simulink model "TosAeb"  (R2021a)
settings: Solver=FixedStepDiscrete  StartTime=0.0  StopTime=inf  FixedStep=0.01  RelTol=1e-3
undefined symbols (not defined in the file or in its folder): ACCModSts, AccSts1, AEB_ObjectMovingOrientation, AEB_ObjectMovingState, CN_BTCActive, CN_CurrentGearR, CN_CurrentGearR_DHT, CN_PTCActive, CN_VDCActive, CN_VSC_EspOff, CN_VSC_FCW_AEB_Error, CN_VSC_FCW_AEB_NoDispay, CN_VSC_FCW_AEB_PerfmDgdtn, CN_VSC_FCW_AEB_TempUnAvlbl, CN_VSC_VehStandstill, CNaccelAvoidMax, CNaccelAvoidMin, CNbrakePedalPress, CNcomfortStrLeftAccelMax, CNcomfortStrLeftAccelMin, CNcomfortStrRightAccelMax, CNcomfortStrRightAccelMin, CNDrvDoorOpen, CNDrvModeEco, CNDrvModeNorm, CNDrvModeOther, CNDrvModeSport, CNDrvSBRreminder, CNNull, CNobjInvalidID, CNPassDoorOpen, CNPassSBRreminder, CNPEBSAccelNegInf, CNPEBSAccelNull, CNPEBSTimeInf, CNPEBSTimeNull, CNSTOPMAX, CNSTOPNULL, CNsubjWidth, CNSwtOFF, CNSwtON, CNTTCMAX, CNTTCNULL, CNVehConfigAEBCycNone, CNVehConfigAEBNone, CNVehConfigAEBPedNone, CNVehConfigFCWNone, CNVehConfigJANone, CollisionSeverity, FALSE, FCWSnvty, JACycObjCalcInfoDefaultParams, JAPedObjCalcInfoDefaultParams, JAVehObjCalcInfoDefaultParams, latCycObjCalcInfoDefaultParams, latPedObjCalcInfoDefaultParams, longObjCalcInfoDefaultParams, ModSts, movingStatus, ObjectType ... (+1112)
libraries: simulink x3031, AEBSFunctionLib x50, Ta_Library x33, AEBSStateMachineLib x23, Mylibrary x12, simulink_extras x7, ObjSelect_lib x3, dspstat3 x2
(part 34 of 102)

== system TosAeb/FcwAebJa_Step_sys/ALG_AEBSystem/ALG_AEBController/AEB_VehicleStateCalcApp/JaSuppAborCondtionCalc/JAVehPrefillSuppAborConditonCalc/VehStateCalcJAPrefillAborCalc ==
  AEB_JAResp : Inport
  FCW_AEB_JAFctSts : Inport  Port=2
  vehAssociatedSysStateSuppCond : Inport  Port=3
  vehUnstableStateFlg : Inport  Port=4
  Compare To Constant : simulink/Logic and Bit Operations/Compare To Constant  relop===  const=CNSwtOFF  OutDataTypeStr=boolean
  Compare To Constant2 : simulink/Logic and Bit Operations/Compare To Constant  relop===  const=TRUE  OutDataTypeStr=boolean
  Compare To Constant3 : simulink/Logic and Bit Operations/Compare To Constant  relop===  const=TRUE  OutDataTypeStr=boolean
  Compare To Constant4 : simulink/Logic and Bit Operations/Compare To Constant  relop===  const=CN_VSC_FCW_AEB_Error  OutDataTypeStr=boolean
  Compare To Constant8 : simulink/Logic and Bit Operations/Compare To Constant  relop===  const=CN_VSC_FCW_AEB_PerfmDgdtn  OutDataTypeStr=boolean
  Compare To Constant9 : simulink/Logic and Bit Operations/Compare To Constant  relop===  const=CN_VSC_FCW_AEB_TempUnAvlbl  OutDataTypeStr=boolean
  Logical Operator : Logic  Operator=OR  Inputs=3  AllPortsSameDT=off  OutDataTypeStr=boolean
  Logical Operator1 : Logic  Operator=OR  Inputs=4  AllPortsSameDT=off  OutDataTypeStr=boolean
  VSCJAVehPrefillAborCond : Outport
  wires:
  Compare To Constant3/1 -> Logical Operator1/4
  Compare To Constant2/1 -> Logical Operator1/3
  AEB_JAResp/1 -> Compare To Constant/1
  Compare To Constant/1 -> Logical Operator1/1
  vehAssociatedSysStateSuppCond/1 -> Compare To Constant2/1
  Logical Operator1/1 -> VSCJAVehPrefillAborCond/1
  vehUnstableStateFlg/1 -> Compare To Constant3/1
  Compare To Constant4/1 -> Logical Operator/1
  Compare To Constant8/1 -> Logical Operator/2
  Compare To Constant9/1 -> Logical Operator/3
  Logical Operator/1 -> Logical Operator1/2
  FCW_AEB_JAFctSts/1 -> Compare To Constant9/1, Compare To Constant8/1, Compare To Constant4/1

== system TosAeb/FcwAebJa_Step_sys/ALG_AEBSystem/ALG_AEBController/AEB_VehicleStateCalcApp/JaSuppAborCondtionCalc/JAVehPrefillSuppAborConditonCalc/VehStateCalcJAPrefillSuppCalc ==
  AEB_JAResp : Inport
  FCW_AEB_JAFctSts : Inport  Port=2
  vehUnstableStateFlg : Inport  Port=3
  vehAssociatedSysStateSuppCond : Inport  Port=4
  "VehConfigAEBJA " : Inport  Port=5
  blockTimeBrakeReady : Inport  Port=6
  Compare To Constant : simulink/Logic and Bit Operations/Compare To Constant  relop===  const=CNSwtOFF  OutDataTypeStr=boolean
  Compare To Constant2 : simulink/Logic and Bit Operations/Compare To Constant  relop===  const=CN_VSC_FCW_AEB_Error  OutDataTypeStr=boolean
  Compare To Constant3 : simulink/Logic and Bit Operations/Compare To Constant  relop===  const=CNVehConfigJANone  OutDataTypeStr=boolean
  Compare To Constant4 : simulink/Logic and Bit Operations/Compare To Constant  relop=>  const=CNNull  OutDataTypeStr=boolean
  Compare To Constant5 : simulink/Logic and Bit Operations/Compare To Constant  relop===  const=TRUE  OutDataTypeStr=boolean
  Compare To Constant6 : simulink/Logic and Bit Operations/Compare To Constant  relop===  const=TRUE  OutDataTypeStr=boolean
  Compare To Constant7 : simulink/Logic and Bit Operations/Compare To Constant  relop===  const=CNSwtOFF  OutDataTypeStr=boolean
  Compare To Constant8 : simulink/Logic and Bit Operations/Compare To Constant  relop===  const=CN_VSC_FCW_AEB_PerfmDgdtn  OutDataTypeStr=boolean
  Compare To Constant9 : simulink/Logic and Bit Operations/Compare To Constant  relop===  const=CN_VSC_FCW_AEB_TempUnAvlbl  OutDataTypeStr=boolean
  Constant : Constant  Value=P_AEB_InterSwtSts4JAVehPrefill
  Logical Operator : Logic  Operator=OR  Inputs=3  AllPortsSameDT=off  OutDataTypeStr=boolean
  Logical Operator1 : Logic  Operator=OR  Inputs=6  AllPortsSameDT=off  OutDataTypeStr=boolean
  Terminator : Terminator
  VSCJAVehPrefillSuppCond : Outport
  wires:
  Compare To Constant7/1 -> Logical Operator1/6
  Constant/1 -> Compare To Constant7/1
  Compare To Constant6/1 -> Logical Operator1/3
  Compare To Constant5/1 -> Logical Operator1/4
  Compare To Constant4/1 -> Terminator/1
  "VehConfigAEBJA "/1 -> Compare To Constant3/1
  Logical Operator1/1 -> VSCJAVehPrefillSuppCond/1
  vehUnstableStateFlg/1 -> Compare To Constant6/1
  blockTimeBrakeReady/1 -> Compare To Constant4/1
  vehAssociatedSysStateSuppCond/1 -> Compare To Constant5/1
  Compare To Constant3/1 -> Logical Operator1/5
  Compare To Constant/1 -> Logical Operator1/1
  AEB_JAResp/1 -> Compare To Constant/1
  Compare To Constant2/1 -> Logical Operator/1
  Compare To Constant8/1 -> Logical Operator/2
  Compare To Constant9/1 -> Logical Operator/3
  Logical Operator/1 -> Logical Operator1/2
  FCW_AEB_JAFctSts/1 -> Compare To Constant9/1, Compare To Constant8/1, Compare To Constant2/1

== system TosAeb/FcwAebJa_Step_sys/ALG_AEBSystem/ALG_AEBController/AEB_VehicleStateCalcApp/PedSuppAborCondtionCalc/PedABASuppAborConditonCalc/VehStateCalcPedABAAborCalc ==
  AEB_PedResp : Inport
  FCW_AEB_PedFctSts : Inport  Port=2
  Compare To Constant : simulink/Logic and Bit Operations/Compare To Constant  relop===  const=CNSwtOFF  OutDataTypeStr=boolean
  Compare To Constant1 : simulink/Logic and Bit Operations/Compare To Constant  relop===  const=CN_VSC_FCW_AEB_Error  OutDataTypeStr=boolean
  Compare To Constant2 : simulink/Logic and Bit Operations/Compare To Constant  relop===  const=CN_VSC_FCW_AEB_PerfmDgdtn  OutDataTypeStr=boolean
  Compare To Constant8 : simulink/Logic and Bit Operations/Compare To Constant  relop===  const=CN_VSC_FCW_AEB_TempUnAvlbl  OutDataTypeStr=boolean
  Logical Operator : Logic  Operator=OR  Inputs=3  AllPortsSameDT=off  OutDataTypeStr=boolean
  Logical Operator1 : Logic  Operator=OR  AllPortsSameDT=off  OutDataTypeStr=boolean
  VSCPedABAAborCond : Outport
  wires:
  FCW_AEB_PedFctSts/1 -> Compare To Constant1/1, Compare To Constant2/1, Compare To Constant8/1
  Compare To Constant8/1 -> Logical Operator/3
  Compare To Constant1/1 -> Logical Operator/1
  Compare To Constant2/1 -> Logical Operator/2
  AEB_PedResp/1 -> Compare To Constant/1
  Compare To Constant/1 -> Logical Operator1/1
  Logical Operator/1 -> Logical Operator1/2
  Logical Operator1/1 -> VSCPedABAAborCond/1

== system TosAeb/FcwAebJa_Step_sys/ALG_AEBSystem/ALG_AEBController/AEB_VehicleStateCalcApp/PedSuppAborCondtionCalc/PedABASuppAborConditonCalc/VehStateCalcPedABASuppCalc ==
  VSCAEB_PedResp : Inport
  FCW_AEB_PedFctSts : Inport  Port=2
  vehUnstableStateFlg : Inport  Port=3
  vehAssociatedSysStateSuppCond : Inport  Port=4
  "vehConfigAEBPed " : Inport  Port=5
  blockTimeBrakeReady : Inport  Port=6
  Compare To Constant : simulink/Logic and Bit Operations/Compare To Constant  relop===  const=CNSwtOFF  OutDataTypeStr=boolean
  Compare To Constant1 : simulink/Logic and Bit Operations/Compare To Constant  relop===  const=CN_VSC_FCW_AEB_Error  OutDataTypeStr=boolean
  Compare To Constant2 : simulink/Logic and Bit Operations/Compare To Constant  relop===  const=TRUE  OutDataTypeStr=boolean
  Compare To Constant3 : simulink/Logic and Bit Operations/Compare To Constant  relop===  const=CNVehConfigAEBPedNone  OutDataTypeStr=boolean
  Compare To Constant4 : simulink/Logic and Bit Operations/Compare To Constant  relop=>  const=CNNull  OutDataTypeStr=boolean
  Compare To Constant5 : simulink/Logic and Bit Operations/Compare To Constant  relop===  const=TRUE  OutDataTypeStr=boolean
  Compare To Constant6 : simulink/Logic and Bit Operations/Compare To Constant  relop===  const=CN_VSC_FCW_AEB_PerfmDgdtn  OutDataTypeStr=boolean
  Compare To Constant7 : simulink/Logic and Bit Operations/Compare To Constant  relop===  const=CNSwtOFF  OutDataTypeStr=boolean
  Compare To Constant8 : simulink/Logic and Bit Operations/Compare To Constant  relop===  const=CN_VSC_FCW_AEB_TempUnAvlbl  OutDataTypeStr=boolean
  Constant : Constant  Value=P_AEB_InterSwtSts4PedABA
  Logical Operator : Logic  Operator=OR  Inputs=3  AllPortsSameDT=off  OutDataTypeStr=boolean
  Logical Operator1 : Logic  Operator=OR  Inputs=6  AllPortsSameDT=off  OutDataTypeStr=boolean
  Terminator : Terminator
  VSCPedABASuppCond : Outport
  wires:
  FCW_AEB_PedFctSts/1 -> Compare To Constant1/1, Compare To Constant6/1, Compare To Constant8/1
  Logical Operator/1 -> Logical Operator1/2
  Compare To Constant8/1 -> Logical Operator/3
  Compare To Constant1/1 -> Logical Operator/1
  Compare To Constant6/1 -> Logical Operator/2
  Compare To Constant7/1 -> Logical Operator1/6
  Constant/1 -> Compare To Constant7/1
  Compare To Constant5/1 -> Logical Operator1/4
  Compare To Constant2/1 -> Logical Operator1/3
  Compare To Constant4/1 -> Terminator/1
  "vehConfigAEBPed "/1 -> Compare To Constant3/1
  Logical Operator1/1 -> VSCPedABASuppCond/1
  vehUnstableStateFlg/1 -> Compare To Constant2/1
  blockTimeBrakeReady/1 -> Compare To Constant4/1
  vehAssociatedSysStateSuppCond/1 -> Compare To Constant5/1
  Compare To Constant3/1 -> Logical Operator1/5
  Compare To Constant/1 -> Logical Operator1/1
  VSCAEB_PedResp/1 -> Compare To Constant/1

== system TosAeb/FcwAebJa_Step_sys/ALG_AEBSystem/ALG_AEBController/AEB_VehicleStateCalcApp/PedSuppAborCondtionCalc/PedAEBfullSuppAborConditonCalc/VehStateCalcPedAEBfullAborCalc ==
  AEB_PedResp : Inport
  FCW_AEB_PedFctSts : Inport  Port=2
  vehAssociatedSysStateSuppCond : Inport  Port=3
  vehUnstableStateFlg : Inport  Port=4
  Compare To Constant : simulink/Logic and Bit Operations/Compare To Constant  relop===  const=CNSwtOFF  OutDataTypeStr=boolean
  Compare To Constant2 : simulink/Logic and Bit Operations/Compare To Constant  relop===  const=TRUE  OutDataTypeStr=boolean
  Compare To Constant3 : simulink/Logic and Bit Operations/Compare To Constant  relop===  const=TRUE  OutDataTypeStr=boolean
  Compare To Constant4 : simulink/Logic and Bit Operations/Compare To Constant  relop===  const=CN_VSC_FCW_AEB_Error  OutDataTypeStr=boolean
  Compare To Constant5 : simulink/Logic and Bit Operations/Compare To Constant  relop===  const=CN_VSC_FCW_AEB_PerfmDgdtn  OutDataTypeStr=boolean
  Compare To Constant8 : simulink/Logic and Bit Operations/Compare To Constant  relop===  const=CN_VSC_FCW_AEB_TempUnAvlbl  OutDataTypeStr=boolean
  Logical Operator : Logic  Operator=OR  Inputs=3  AllPortsSameDT=off  OutDataTypeStr=boolean
  Logical Operator1 : Logic  Operator=OR  Inputs=4  AllPortsSameDT=off  OutDataTypeStr=boolean
  VSCPedAEBfullAborCond : Outport
  wires:
  FCW_AEB_PedFctSts/1 -> Compare To Constant4/1, Compare To Constant5/1, Compare To Constant8/1
  Compare To Constant4/1 -> Logical Operator/1
  Compare To Constant5/1 -> Logical Operator/2
  Compare To Constant8/1 -> Logical Operator/3
  Compare To Constant3/1 -> Logical Operator1/4
  Compare To Constant2/1 -> Logical Operator1/3
  vehUnstableStateFlg/1 -> Compare To Constant3/1
  Logical Operator1/1 -> VSCPedAEBfullAborCond/1
  Logical Operator/1 -> Logical Operator1/2
  vehAssociatedSysStateSuppCond/1 -> Compare To Constant2/1
  Compare To Constant/1 -> Logical Operator1/1
  AEB_PedResp/1 -> Compare To Constant/1

== system TosAeb/FcwAebJa_Step_sys/ALG_AEBSystem/ALG_AEBController/AEB_VehicleStateCalcApp/PedSuppAborCondtionCalc/PedAEBfullSuppAborConditonCalc/VehStateCalcPedAEBfullSuppCalc ==
  AEB_PedResp : Inport
  FCW_AEB_PedFctSts : Inport  Port=2
  vehUnstableStateFlg : Inport  Port=3
  vehAssociatedSysStateSuppCond : Inport  Port=4
  "vehConfigAEBPed " : Inport  Port=5
  blockTimeBrake : Inport  Port=6
  Compare To Constant : simulink/Logic and Bit Operations/Compare To Constant  relop===  const=CNSwtOFF  OutDataTypeStr=boolean
  Compare To Constant1 : simulink/Logic and Bit Operations/Compare To Constant  relop===  const=CN_VSC_FCW_AEB_Error  OutDataTypeStr=boolean
  Compare To Constant2 : simulink/Logic and Bit Operations/Compare To Constant  relop===  const=TRUE  OutDataTypeStr=boolean
  Compare To Constant3 : simulink/Logic and Bit Operations/Compare To Constant  relop===  const=CNVehConfigAEBPedNone  OutDataTypeStr=boolean
  Compare To Constant4 : simulink/Logic and Bit Operations/Compare To Constant  relop=>  const=CNNull  OutDataTypeStr=boolean
  Compare To Constant5 : simulink/Logic and Bit Operations/Compare To Constant  relop===  const=TRUE  OutDataTypeStr=boolean
  Compare To Constant6 : simulink/Logic and Bit Operations/Compare To Constant  relop===  const=CN_VSC_FCW_AEB_PerfmDgdtn  OutDataTypeStr=boolean
  Compare To Constant7 : simulink/Logic and Bit Operations/Compare To Constant  relop===  const=CNSwtOFF  OutDataTypeStr=boolean
  Compare To Constant8 : simulink/Logic and Bit Operations/Compare To Constant  relop===  const=CN_VSC_FCW_AEB_TempUnAvlbl  OutDataTypeStr=boolean
  Constant : Constant  Value=P_AEB_InterSwtSts4PedAEBfull
  Logical Operator : Logic  Operator=OR  Inputs=3  AllPortsSameDT=off  OutDataTypeStr=boolean
  Logical Operator1 : Logic  Operator=OR  Inputs=7  AllPortsSameDT=off  OutDataTypeStr=boolean
  VSCPedAEBfullSuppCond : Outport
  wires:
  FCW_AEB_PedFctSts/1 -> Compare To Constant1/1, Compare To Constant6/1, Compare To Constant8/1
  Compare To Constant1/1 -> Logical Operator/1
  Compare To Constant6/1 -> Logical Operator/2
  Compare To Constant8/1 -> Logical Operator/3
  Compare To Constant7/1 -> Logical Operator1/7
  Constant/1 -> Compare To Constant7/1
  Compare To Constant5/1 -> Logical Operator1/4
  Compare To Constant2/1 -> Logical Operator1/3
  AEB_PedResp/1 -> Compare To Constant/1
  Compare To Constant/1 -> Logical Operator1/1
  Compare To Constant3/1 -> Logical Operator1/5
  vehAssociatedSysStateSuppCond/1 -> Compare To Constant5/1
  blockTimeBrake/1 -> Compare To Constant4/1
  Logical Operator/1 -> Logical Operator1/2
  vehUnstableStateFlg/1 -> Compare To Constant2/1
  Logical Operator1/1 -> VSCPedAEBfullSuppCond/1
  "vehConfigAEBPed "/1 -> Compare To Constant3/1
  Compare To Constant4/1 -> Logical Operator1/6

== system TosAeb/FcwAebJa_Step_sys/ALG_AEBSystem/ALG_AEBController/AEB_VehicleStateCalcApp/PedSuppAborCondtionCalc/PedPreWaringSuppAborConditonCalc/VehStateCalcPedPreWaringAborCalc ==
  VSCAEB_PedResp : Inport
  FCW_AEB_PedFctSts : Inport  Port=2
  vehAssociatedSysStateSuppCond : Inport  Port=3
  Compare To Constant : simulink/Logic and Bit Operations/Compare To Constant  relop===  const=CNSwtOFF  OutDataTypeStr=boolean
  Compare To Constant1 : simulink/Logic and Bit Operations/Compare To Constant  relop===  const=CN_VSC_FCW_AEB_Error  OutDataTypeStr=boolean
  Compare To Constant2 : simulink/Logic and Bit Operations/Compare To Constant  relop===  const=TRUE  OutDataTypeStr=boolean
  Compare To Constant3 : simulink/Logic and Bit Operations/Compare To Constant  relop===  const=CN_VSC_FCW_AEB_PerfmDgdtn  OutDataTypeStr=boolean
  Compare To Constant8 : simulink/Logic and Bit Operations/Compare To Constant  relop===  const=CN_VSC_FCW_AEB_TempUnAvlbl  OutDataTypeStr=boolean
  Logical Operator : Logic  Operator=OR  Inputs=3  AllPortsSameDT=off  OutDataTypeStr=boolean
  Logical Operator1 : Logic  Operator=OR  Inputs=3  AllPortsSameDT=off  OutDataTypeStr=boolean
  VSCPedPreWaringAborCond : Outport
  wires:
  FCW_AEB_PedFctSts/1 -> Compare To Constant1/1, Compare To Constant3/1, Compare To Constant8/1
  Compare To Constant1/1 -> Logical Operator/1
  Compare To Constant3/1 -> Logical Operator/2
  Compare To Constant8/1 -> Logical Operator/3
  Compare To Constant2/1 -> Logical Operator1/3
  VSCAEB_PedResp/1 -> Compare To Constant/1
  Compare To Constant/1 -> Logical Operator1/1
  vehAssociatedSysStateSuppCond/1 -> Compare To Constant2/1
  Logical Operator/1 -> Logical Operator1/2
  Logical Operator1/1 -> VSCPedPreWaringAborCond/1

== system TosAeb/FcwAebJa_Step_sys/ALG_AEBSystem/ALG_AEBController/AEB_VehicleStateCalcApp/PedSuppAborCondtionCalc/PedPreWaringSuppAborConditonCalc/VehStateCalcPedPreWaringSuppCalc ==
  AEB_PedResp : Inport
  FCW_AEB_PedFctSts : Inport  Port=2
  vehUnstableStateFlg : Inport  Port=3
  vehAssociatedSysStateSuppCond : Inport  Port=4
  "vehConfigAEBPed " : Inport  Port=5
  blockTimeWarning : Inport  Port=6
  Compare To Constant : simulink/Logic and Bit Operations/Compare To Constant  relop===  const=CNSwtOFF  OutDataTypeStr=boolean
  Compare To Constant1 : simulink/Logic and Bit Operations/Compare To Constant  relop===  const=CN_VSC_FCW_AEB_Error  OutDataTypeStr=boolean
  Compare To Constant2 : simulink/Logic and Bit Operations/Compare To Constant  relop===  const=TRUE  OutDataTypeStr=boolean
  Compare To Constant3 : simulink/Logic and Bit Operations/Compare To Constant  relop===  const=CNVehConfigAEBPedNone  OutDataTypeStr=boolean
  Compare To Constant4 : simulink/Logic and Bit Operations/Compare To Constant  relop=>  const=CNNull  OutDataTypeStr=boolean
  Compare To Constant5 : simulink/Logic and Bit Operations/Compare To Constant  relop===  const=TRUE  OutDataTypeStr=boolean
  Compare To Constant6 : simulink/Logic and Bit Operations/Compare To Constant  relop===  const=CN_VSC_FCW_AEB_PerfmDgdtn  OutDataTypeStr=boolean
  Compare To Constant7 : simulink/Logic and Bit Operations/Compare To Constant  relop===  const=CNSwtOFF  OutDataTypeStr=boolean
  Compare To Constant8 : simulink/Logic and Bit Operations/Compare To Constant  relop===  const=CN_VSC_FCW_AEB_TempUnAvlbl  OutDataTypeStr=boolean
  Constant : Constant  Value=P_AEB_InterSwtSts4PedPreWaring
  Logical Operator : Logic  Operator=OR  Inputs=3  AllPortsSameDT=off  OutDataTypeStr=boolean
  Logical Operator1 : Logic  Operator=OR  Inputs=7  AllPortsSameDT=off  OutDataTypeStr=boolean
  VSCPedPreWaringSuppCond : Outport
  wires:
  FCW_AEB_PedFctSts/1 -> Compare To Constant1/1, Compare To Constant6/1, Compare To Constant8/1
  Compare To Constant1/1 -> Logical Operator/1
  Compare To Constant6/1 -> Logical Operator/2
  Compare To Constant8/1 -> Logical Operator/3
  Compare To Constant7/1 -> Logical Operator1/7
  Constant/1 -> Compare To Constant7/1
  Compare To Constant5/1 -> Logical Operator1/3
  Compare To Constant2/1 -> Logical Operator1/4
  Compare To Constant4/1 -> Logical Operator1/6
  "vehConfigAEBPed "/1 -> Compare To Constant3/1
  Logical Operator1/1 -> VSCPedPreWaringSuppCond/1
  vehUnstableStateFlg/1 -> Compare To Constant5/1
  Logical Operator/1 -> Logical Operator1/2
  blockTimeWarning/1 -> Compare To Constant4/1
  vehAssociatedSysStateSuppCond/1 -> Compare To Constant2/1
  Compare To Constant3/1 -> Logical Operator1/5
  Compare To Constant/1 -> Logical Operator1/1
  AEB_PedResp/1 -> Compare To Constant/1

== system TosAeb/FcwAebJa_Step_sys/ALG_AEBSystem/ALG_AEBController/AEB_VehicleStateCalcApp/PedSuppAborCondtionCalc/PedPrefillSuppAborConditonCalc/VehStateCalcPrefillAborCalc ==
  AEB_PedResp : Inport
  FCW_AEB_PedFctSts : Inport  Port=2
  vehUnstableStateFlg : Inport  Port=3
  vehAssociatedSysStateSuppCond : Inport  Port=4
  Compare To Constant : simulink/Logic and Bit Operations/Compare To Constant  relop===  const=CNSwtOFF  OutDataTypeStr=boolean
  Compare To Constant2 : simulink/Logic and Bit Operations/Compare To Constant  relop===  const=TRUE  OutDataTypeStr=boolean
  Compare To Constant3 : simulink/Logic and Bit Operations/Compare To Constant  relop===  const=TRUE  OutDataTypeStr=boolean
  Compare To Constant4 : simulink/Logic and Bit Operations/Compare To Constant  relop===  const=CN_VSC_FCW_AEB_Error  OutDataTypeStr=boolean
  Compare To Constant5 : simulink/Logic and Bit Operations/Compare To Constant  relop===  const=CN_VSC_FCW_AEB_PerfmDgdtn  OutDataTypeStr=boolean
  Compare To Constant8 : simulink/Logic and Bit Operations/Compare To Constant  relop===  const=CN_VSC_FCW_AEB_TempUnAvlbl  OutDataTypeStr=boolean
  Logical Operator : Logic  Operator=OR  Inputs=3  AllPortsSameDT=off  OutDataTypeStr=boolean
  Logical Operator1 : Logic  Operator=OR  Inputs=4  AllPortsSameDT=off  OutDataTypeStr=boolean
  VSCPedPrefillAborCond : Outport
  wires:
  FCW_AEB_PedFctSts/1 -> Compare To Constant4/1, Compare To Constant5/1, Compare To Constant8/1
  Logical Operator/1 -> Logical Operator1/2
  Compare To Constant8/1 -> Logical Operator/3
  Compare To Constant4/1 -> Logical Operator/1
  Compare To Constant5/1 -> Logical Operator/2
  Compare To Constant3/1 -> Logical Operator1/4
  Compare To Constant2/1 -> Logical Operator1/3
  Logical Operator1/1 -> VSCPedPrefillAborCond/1
  vehUnstableStateFlg/1 -> Compare To Constant2/1
  vehAssociatedSysStateSuppCond/1 -> Compare To Constant3/1
  Compare To Constant/1 -> Logical Operator1/1
  AEB_PedResp/1 -> Compare To Constant/1

== system TosAeb/FcwAebJa_Step_sys/ALG_AEBSystem/ALG_AEBController/AEB_VehicleStateCalcApp/PedSuppAborCondtionCalc/PedPrefillSuppAborConditonCalc/VehStateCalcPrefillSuppCalc ==
  AEB_PedResp : Inport
  FCW_AEB_PedFctSts : Inport  Port=2
  vehUnstableStateFlg : Inport  Port=3
  vehAssociatedSysStateSuppCond : Inport  Port=4
  "vehConfigAEBPed " : Inport  Port=5
  blockTimeBrakeReady : Inport  Port=6
  Compare To Constant : simulink/Logic and Bit Operations/Compare To Constant  relop===  const=CNSwtOFF  OutDataTypeStr=boolean
  Compare To Constant2 : simulink/Logic and Bit Operations/Compare To Constant  relop===  const=CN_VSC_FCW_AEB_Error  OutDataTypeStr=boolean
  Compare To Constant3 : simulink/Logic and Bit Operations/Compare To Constant  relop===  const=CNVehConfigAEBPedNone  OutDataTypeStr=boolean
  Compare To Constant4 : simulink/Logic and Bit Operations/Compare To Constant  relop=>  const=CNNull  OutDataTypeStr=boolean
  Compare To Constant5 : simulink/Logic and Bit Operations/Compare To Constant  relop===  const=TRUE  OutDataTypeStr=boolean
  Compare To Constant6 : simulink/Logic and Bit Operations/Compare To Constant  relop===  const=TRUE  OutDataTypeStr=boolean
  Compare To Constant7 : simulink/Logic and Bit Operations/Compare To Constant  relop===  const=CNSwtOFF  OutDataTypeStr=boolean
  Compare To Constant8 : simulink/Logic and Bit Operations/Compare To Constant  relop===  const=CN_VSC_FCW_AEB_PerfmDgdtn  OutDataTypeStr=boolean
  Compare To Constant9 : simulink/Logic and Bit Operations/Compare To Constant  relop===  const=CN_VSC_FCW_AEB_TempUnAvlbl  OutDataTypeStr=boolean
  Constant : Constant  Value=P_AEB_InterSwtSts4PedPrefill
  Logical Operator : Logic  Operator=OR  Inputs=3  AllPortsSameDT=off  OutDataTypeStr=boolean
  Logical Operator1 : Logic  Operator=OR  Inputs=6  AllPortsSameDT=off  OutDataTypeStr=boolean
  Terminator : Terminator
  VSCPedPrefillSuppCond : Outport
  wires:
  FCW_AEB_PedFctSts/1 -> Compare To Constant9/1, Compare To Constant8/1, Compare To Constant2/1
  Logical Operator/1 -> Logical Operator1/2
  Compare To Constant9/1 -> Logical Operator/3
  Compare To Constant2/1 -> Logical Operator/1
  Compare To Constant8/1 -> Logical Operator/2
  Compare To Constant7/1 -> Logical Operator1/6
  Constant/1 -> Compare To Constant7/1
  Compare To Constant6/1 -> Logical Operator1/3
  Compare To Constant5/1 -> Logical Operator1/4
  AEB_PedResp/1 -> Compare To Constant/1
  Compare To Constant/1 -> Logical Operator1/1
  Compare To Constant3/1 -> Logical Operator1/5
  vehAssociatedSysStateSuppCond/1 -> Compare To Constant5/1
  blockTimeBrakeReady/1 -> Compare To Constant4/1
  vehUnstableStateFlg/1 -> Compare To Constant6/1
  Logical Operator1/1 -> VSCPedPrefillSuppCond/1
  "vehConfigAEBPed "/1 -> Compare To Constant3/1
  Compare To Constant4/1 -> Terminator/1

== system TosAeb/FcwAebJa_Step_sys/ALG_AEBSystem/ALG_AEBController/AEB_VehicleStateCalcApp/VehSuppAborCondtionCalc/ABASuppAborConditonCalc/VehStateCalcABAAborCalc ==
  AEB_VehResp : Inport
  FCW_AEB_VehFctSts : Inport  Port=2
  Compare To Constant : simulink/Logic and Bit Operations/Compare To Constant  relop===  const=CNSwtOFF  OutDataTypeStr=boolean
  Compare To Constant1 : simulink/Logic and Bit Operations/Compare To Constant  relop===  const=CN_VSC_FCW_AEB_Error  OutDataTypeStr=boolean
  Compare To Constant2 : simulink/Logic and Bit Operations/Compare To Constant  relop===  const=CN_VSC_FCW_AEB_PerfmDgdtn  OutDataTypeStr=boolean
  Compare To Constant8 : simulink/Logic and Bit Operations/Compare To Constant  relop===  const=CN_VSC_FCW_AEB_TempUnAvlbl  OutDataTypeStr=boolean
  Logical Operator : Logic  Operator=OR  Inputs=3  AllPortsSameDT=off  OutDataTypeStr=boolean
  Logical Operator1 : Logic  Operator=OR  AllPortsSameDT=off  OutDataTypeStr=boolean
  VSCvehABAAborCond : Outport
  wires:
  Compare To Constant8/1 -> Logical Operator/3
  Compare To Constant1/1 -> Logical Operator/1
  Compare To Constant2/1 -> Logical Operator/2
  AEB_VehResp/1 -> Compare To Constant/1
  Compare To Constant/1 -> Logical Operator1/1
  FCW_AEB_VehFctSts/1 -> Compare To Constant1/1, Compare To Constant2/1, Compare To Constant8/1
  Logical Operator/1 -> Logical Operator1/2
  Logical Operator1/1 -> VSCvehABAAborCond/1

== system TosAeb/FcwAebJa_Step_sys/ALG_AEBSystem/ALG_AEBController/AEB_VehicleStateCalcApp/VehSuppAborCondtionCalc/ABASuppAborConditonCalc/VehStateCalcABAtSuppCalc ==
  AEB_VehResp : Inport
  FCW_AEB_VehFctSts : Inport  Port=2
  vehUnstableStateFlg : Inport  Port=3
  vehAssociatedSysStateSuppCond : Inport  Port=4
  "vehConfigAEB " : Inport  Port=5
  blockTimeBrakeReady : Inport  Port=6
  Compare To Constant : simulink/Logic and Bit Operations/Compare To Constant  relop===  const=CNSwtOFF  OutDataTypeStr=boolean
  Compare To Constant1 : simulink/Logic and Bit Operations/Compare To Constant  relop===  const=CN_VSC_FCW_AEB_Error  OutDataTypeStr=boolean
  Compare To Constant2 : simulink/Logic and Bit Operations/Compare To Constant  relop===  const=CN_VSC_FCW_AEB_PerfmDgdtn  OutDataTypeStr=boolean
  Compare To Constant3 : simulink/Logic and Bit Operations/Compare To Constant  relop===  const=CNVehConfigAEBNone  OutDataTypeStr=boolean
  Compare To Constant4 : simulink/Logic and Bit Operations/Compare To Constant  relop=>  const=CNNull  OutDataTypeStr=boolean
  Compare To Constant5 : simulink/Logic and Bit Operations/Compare To Constant  relop===  const=TRUE  OutDataTypeStr=boolean
  Compare To Constant6 : simulink/Logic and Bit Operations/Compare To Constant  relop===  const=TRUE  OutDataTypeStr=boolean
  Compare To Constant7 : simulink/Logic and Bit Operations/Compare To Constant  relop===  const=CNSwtOFF  OutDataTypeStr=boolean
  Compare To Constant8 : simulink/Logic and Bit Operations/Compare To Constant  relop===  const=CN_VSC_FCW_AEB_TempUnAvlbl  OutDataTypeStr=boolean
  Constant : Constant  Value=P_AEB_InterSwtSts4ABA
  Logical Operator : Logic  Operator=OR  Inputs=3  AllPortsSameDT=off  OutDataTypeStr=boolean
  Logical Operator1 : Logic  Operator=OR  Inputs=6  AllPortsSameDT=off  OutDataTypeStr=boolean
  Terminator : Terminator
  VSCvehABASuppCond : Outport
  wires:
  Compare To Constant8/1 -> Logical Operator/3
  Compare To Constant1/1 -> Logical Operator/1
  Compare To Constant2/1 -> Logical Operator/2
  Compare To Constant7/1 -> Logical Operator1/6
  Constant/1 -> Compare To Constant7/1
  Compare To Constant6/1 -> Logical Operator1/3
  Compare To Constant5/1 -> Logical Operator1/4
  Compare To Constant4/1 -> Terminator/1
  "vehConfigAEB "/1 -> Compare To Constant3/1
  Logical Operator1/1 -> VSCvehABASuppCond/1
  vehUnstableStateFlg/1 -> Compare To Constant6/1
  Logical Operator/1 -> Logical Operator1/2
  blockTimeBrakeReady/1 -> Compare To Constant4/1
  FCW_AEB_VehFctSts/1 -> Compare To Constant1/1, Compare To Constant2/1, Compare To Constant8/1
  vehAssociatedSysStateSuppCond/1 -> Compare To Constant5/1
  Compare To Constant3/1 -> Logical Operator1/5
  Compare To Constant/1 -> Logical Operator1/1
  AEB_VehResp/1 -> Compare To Constant/1

== system TosAeb/FcwAebJa_Step_sys/ALG_AEBSystem/ALG_AEBController/AEB_VehicleStateCalcApp/VehSuppAborCondtionCalc/AEBfullSuppAborConditonCalc/VehStateCalcAEBfullAborCalc ==
  AEB_VehResp : Inport
  FCW_AEB_VehFctSts : Inport  Port=2
  vehAssociatedSysStateSuppCond : Inport  Port=3
  vehUnstableStateFlg : Inport  Port=4
  Compare To Constant : simulink/Logic and Bit Operations/Compare To Constant  relop===  const=CNSwtOFF  OutDataTypeStr=boolean
  Compare To Constant1 : simulink/Logic and Bit Operations/Compare To Constant  relop===  const=CN_VSC_FCW_AEB_Error  OutDataTypeStr=boolean
  Compare To Constant2 : simulink/Logic and Bit Operations/Compare To Constant  relop===  const=CN_VSC_FCW_AEB_PerfmDgdtn  OutDataTypeStr=boolean
  Compare To Constant3 : simulink/Logic and Bit Operations/Compare To Constant  relop===  const=TRUE  OutDataTypeStr=boolean
  Compare To Constant7 : simulink/Logic and Bit Operations/Compare To Constant  relop===  const=TRUE  OutDataTypeStr=boolean
  Compare To Constant8 : simulink/Logic and Bit Operations/Compare To Constant  relop===  const=CN_VSC_FCW_AEB_TempUnAvlbl  OutDataTypeStr=boolean
  Logical Operator : Logic  Operator=OR  Inputs=3  AllPortsSameDT=off  OutDataTypeStr=boolean
  Logical Operator1 : Logic  Operator=OR  Inputs=4  AllPortsSameDT=off  OutDataTypeStr=boolean
  VSCvehAEBfullAborCond : Outport
  wires:
  FCW_AEB_VehFctSts/1 -> Compare To Constant1/1, Compare To Constant2/1, Compare To Constant8/1
  Compare To Constant8/1 -> Logical Operator/3
  Compare To Constant1/1 -> Logical Operator/1
  Compare To Constant2/1 -> Logical Operator/2
  Compare To Constant3/1 -> Logical Operator1/4
  Compare To Constant7/1 -> Logical Operator1/3
  AEB_VehResp/1 -> Compare To Constant/1
  Compare To Constant/1 -> Logical Operator1/1
  Logical Operator/1 -> Logical Operator1/2
  Logical Operator1/1 -> VSCvehAEBfullAborCond/1
  vehAssociatedSysStateSuppCond/1 -> Compare To Constant7/1
  vehUnstableStateFlg/1 -> Compare To Constant3/1

== system TosAeb/FcwAebJa_Step_sys/ALG_AEBSystem/ALG_AEBController/AEB_VehicleStateCalcApp/VehSuppAborCondtionCalc/AEBfullSuppAborConditonCalc/VehStateCalcAEBfullSuppCalc ==
  AEB_VehResp : Inport
  FCW_AEB_VehFctSts : Inport  Port=2
  vehUnstableStateFlg : Inport  Port=3
  vehAssociatedSysStateSuppCond : Inport  Port=4
  "vehConfigAEB " : Inport  Port=5
  blockTimeBrake : Inport  Port=6
  Compare To Constant : simulink/Logic and Bit Operations/Compare To Constant  relop===  const=CNSwtOFF  OutDataTypeStr=boolean
  Compare To Constant1 : simulink/Logic and Bit Operations/Compare To Constant  relop===  const=CN_VSC_FCW_AEB_Error  OutDataTypeStr=boolean
  Compare To Constant2 : simulink/Logic and Bit Operations/Compare To Constant  relop===  const=CN_VSC_FCW_AEB_PerfmDgdtn  OutDataTypeStr=boolean
  Compare To Constant3 : simulink/Logic and Bit Operations/Compare To Constant  relop===  const=CNVehConfigAEBNone  OutDataTypeStr=boolean
  Compare To Constant4 : simulink/Logic and Bit Operations/Compare To Constant  relop=>  const=CNNull  OutDataTypeStr=boolean
  Compare To Constant5 : simulink/Logic and Bit Operations/Compare To Constant  relop===  const=TRUE  OutDataTypeStr=boolean
  Compare To Constant6 : simulink/Logic and Bit Operations/Compare To Constant  relop===  const=TRUE  OutDataTypeStr=boolean
  Compare To Constant7 : simulink/Logic and Bit Operations/Compare To Constant  relop===  const=CNSwtOFF  OutDataTypeStr=boolean
  Compare To Constant8 : simulink/Logic and Bit Operations/Compare To Constant  relop===  const=CN_VSC_FCW_AEB_TempUnAvlbl  OutDataTypeStr=boolean
  Constant : Constant  Value=P_AEB_InterSwtSts4AEBfull
  Logical Operator : Logic  Operator=OR  Inputs=3  AllPortsSameDT=off  OutDataTypeStr=boolean
  Logical Operator1 : Logic  Operator=OR  Inputs=7  AllPortsSameDT=off  OutDataTypeStr=boolean
  VSCvehAEBfullSuppCond : Outport
  wires:
  Compare To Constant8/1 -> Logical Operator/3
  Compare To Constant1/1 -> Logical Operator/1
  Compare To Constant2/1 -> Logical Operator/2
  Compare To Constant7/1 -> Logical Operator1/7
  Constant/1 -> Compare To Constant7/1
  Compare To Constant6/1 -> Logical Operator1/3
  Compare To Constant5/1 -> Logical Operator1/4
  Compare To Constant4/1 -> Logical Operator1/6
  "vehConfigAEB "/1 -> Compare To Constant3/1
  Logical Operator1/1 -> VSCvehAEBfullSuppCond/1
  vehUnstableStateFlg/1 -> Compare To Constant6/1
  Logical Operator/1 -> Logical Operator1/2
  blockTimeBrake/1 -> Compare To Constant4/1
  FCW_AEB_VehFctSts/1 -> Compare To Constant1/1, Compare To Constant2/1, Compare To Constant8/1
  vehAssociatedSysStateSuppCond/1 -> Compare To Constant5/1
  Compare To Constant3/1 -> Logical Operator1/5
  Compare To Constant/1 -> Logical Operator1/1
  AEB_VehResp/1 -> Compare To Constant/1

== system TosAeb/FcwAebJa_Step_sys/ALG_AEBSystem/ALG_AEBController/AEB_VehicleStateCalcApp/VehSuppAborCondtionCalc/AEBpartSuppAborConditonCalc/VehStateCalcAEBpartAborCalc ==
  AEB_VehResp : Inport
  FCW_AEB_VehFctSts : Inport  Port=2
  vehAssociatedSysStateSuppCond : Inport  Port=3
  vehUnstableStateFlg : Inport  Port=4
  Compare To Constant : simulink/Logic and Bit Operations/Compare To Constant  relop===  const=CNSwtOFF  OutDataTypeStr=boolean
  Compare To Constant1 : simulink/Logic and Bit Operations/Compare To Constant  relop===  const=CN_VSC_FCW_AEB_Error  OutDataTypeStr=boolean
  Compare To Constant2 : simulink/Logic and Bit Operations/Compare To Constant  relop===  const=CN_VSC_FCW_AEB_PerfmDgdtn  OutDataTypeStr=boolean
  Compare To Constant3 : simulink/Logic and Bit Operations/Compare To Constant  relop===  const=TRUE  OutDataTypeStr=boolean
  Compare To Constant4 : simulink/Logic and Bit Operations/Compare To Constant  relop===  const=TRUE  OutDataTypeStr=boolean
  Compare To Constant8 : simulink/Logic and Bit Operations/Compare To Constant  relop===  const=CN_VSC_FCW_AEB_TempUnAvlbl  OutDataTypeStr=boolean
  Logical Operator : Logic  Operator=OR  Inputs=3  AllPortsSameDT=off  OutDataTypeStr=boolean
  Logical Operator1 : Logic  Operator=OR  Inputs=4  AllPortsSameDT=off  OutDataTypeStr=boolean
  VSCvehAEBpartAborCond : Outport
  wires:
  Compare To Constant8/1 -> Logical Operator/3
  Compare To Constant1/1 -> Logical Operator/1
  Compare To Constant2/1 -> Logical Operator/2
  Compare To Constant3/1 -> Logical Operator1/3
  Compare To Constant4/1 -> Logical Operator1/4
  vehAssociatedSysStateSuppCond/1 -> Compare To Constant3/1
  vehUnstableStateFlg/1 -> Compare To Constant4/1
  Logical Operator1/1 -> VSCvehAEBpartAborCond/1
  Logical Operator/1 -> Logical Operator1/2
  FCW_AEB_VehFctSts/1 -> Compare To Constant1/1, Compare To Constant2/1, Compare To Constant8/1
  Compare To Constant/1 -> Logical Operator1/1
  AEB_VehResp/1 -> Compare To Constant/1

== system TosAeb/FcwAebJa_Step_sys/ALG_AEBSystem/ALG_AEBController/AEB_VehicleStateCalcApp/VehSuppAborCondtionCalc/AEBpartSuppAborConditonCalc/VehStateCalcAEBpartSuppCalc ==
  AEB_VehResp : Inport
  FCW_AEB_VehFctSts : Inport  Port=2
  vehUnstableStateFlg : Inport  Port=3
  vehAssociatedSysStateSuppCond : Inport  Port=4
  "vehConfigAEB " : Inport  Port=5
  blockTimeBrake : Inport  Port=6
  Compare To Constant : simulink/Logic and Bit Operations/Compare To Constant  relop===  const=CNSwtOFF  OutDataTypeStr=boolean
  Compare To Constant1 : simulink/Logic and Bit Operations/Compare To Constant  relop===  const=CN_VSC_FCW_AEB_Error  OutDataTypeStr=boolean
  Compare To Constant2 : simulink/Logic and Bit Operations/Compare To Constant  relop===  const=CN_VSC_FCW_AEB_PerfmDgdtn  OutDataTypeStr=boolean
  Compare To Constant3 : simulink/Logic and Bit Operations/Compare To Constant  relop===  const=CNVehConfigAEBNone  OutDataTypeStr=boolean
  Compare To Constant4 : simulink/Logic and Bit Operations/Compare To Constant  relop=>  const=CNNull  OutDataTypeStr=boolean
  Compare To Constant5 : simulink/Logic and Bit Operations/Compare To Constant  relop===  const=TRUE  OutDataTypeStr=boolean
  Compare To Constant6 : simulink/Logic and Bit Operations/Compare To Constant  relop===  const=TRUE  OutDataTypeStr=boolean
  Compare To Constant7 : simulink/Logic and Bit Operations/Compare To Constant  relop===  const=CNSwtOFF  OutDataTypeStr=boolean
  Compare To Constant8 : simulink/Logic and Bit Operations/Compare To Constant  relop===  const=CN_VSC_FCW_AEB_TempUnAvlbl  OutDataTypeStr=boolean
  Constant : Constant  Value=P_AEB_InterSwtSts4AEBpart
  Logical Operator : Logic  Operator=OR  Inputs=3  AllPortsSameDT=off  OutDataTypeStr=boolean
  Logical Operator1 : Logic  Operator=OR  Inputs=7  AllPortsSameDT=off  OutDataTypeStr=boolean
  VSCvehAEBpartSuppCond : Outport
  wires:
  Compare To Constant8/1 -> Logical Operator/3
  Compare To Constant1/1 -> Logical Operator/1
  Compare To Constant2/1 -> Logical Operator/2
  Compare To Constant7/1 -> Logical Operator1/7
  Constant/1 -> Compare To Constant7/1
  Compare To Constant6/1 -> Logical Operator1/3
  Compare To Constant5/1 -> Logical Operator1/4
  AEB_VehResp/1 -> Compare To Constant/1
  Compare To Constant/1 -> Logical Operator1/1
  Compare To Constant3/1 -> Logical Operator1/5
  vehAssociatedSysStateSuppCond/1 -> Compare To Constant5/1
  FCW_AEB_VehFctSts/1 -> Compare To Constant1/1, Compare To Constant2/1, Compare To Constant8/1
  blockTimeBrake/1 -> Compare To Constant4/1
  Logical Operator/1 -> Logical Operator1/2
  vehUnstableStateFlg/1 -> Compare To Constant6/1
  Logical Operator1/1 -> VSCvehAEBpartSuppCond/1
  "vehConfigAEB "/1 -> Compare To Constant3/1
  Compare To Constant4/1 -> Logical Operator1/6

== system TosAeb/FcwAebJa_Step_sys/ALG_AEBSystem/ALG_AEBController/AEB_VehicleStateCalcApp/VehSuppAborCondtionCalc/AcuteWarningSuppAborConditonCalc/VehStateCalcAcuteWarningAborCalc ==
  AEB_VehResp : Inport
  FCW_AEB_VehFctSts : Inport  Port=2
  vehAssociatedSysStateSuppCond : Inport  Port=3
  Compare To Constant : simulink/Logic and Bit Operations/Compare To Constant  relop===  const=CNSwtOFF  OutDataTypeStr=boolean
  Compare To Constant1 : simulink/Logic and Bit Operations/Compare To Constant  relop===  const=CN_VSC_FCW_AEB_Error  OutDataTypeStr=boolean
  Compare To Constant2 : simulink/Logic and Bit Operations/Compare To Constant  relop===  const=CN_VSC_FCW_AEB_PerfmDgdtn  OutDataTypeStr=boolean
  Compare To Constant5 : simulink/Logic and Bit Operations/Compare To Constant  relop===  const=TRUE  OutDataTypeStr=boolean
  Compare To Constant8 : simulink/Logic and Bit Operations/Compare To Constant  relop===  const=CN_VSC_FCW_AEB_TempUnAvlbl  OutDataTypeStr=boolean
  Logical Operator : Logic  Operator=OR  Inputs=3  AllPortsSameDT=off  OutDataTypeStr=boolean
  Logical Operator1 : Logic  Operator=OR  Inputs=3  AllPortsSameDT=off  OutDataTypeStr=boolean
  VSCvehAcuteWarningAborCond : Outport
  wires:
  Compare To Constant8/1 -> Logical Operator/3
  Compare To Constant1/1 -> Logical Operator/1
  Compare To Constant2/1 -> Logical Operator/2
  Compare To Constant5/1 -> Logical Operator1/3
  AEB_VehResp/1 -> Compare To Constant/1
  Compare To Constant/1 -> Logical Operator1/1
  vehAssociatedSysStateSuppCond/1 -> Compare To Constant5/1
  FCW_AEB_VehFctSts/1 -> Compare To Constant1/1, Compare To Constant2/1, Compare To Constant8/1
  Logical Operator/1 -> Logical Operator1/2
  Logical Operator1/1 -> VSCvehAcuteWarningAborCond/1

== system TosAeb/FcwAebJa_Step_sys/ALG_AEBSystem/ALG_AEBController/AEB_VehicleStateCalcApp/VehSuppAborCondtionCalc/AcuteWarningSuppAborConditonCalc/VehStateCalcAcuteWarningSuppCalc ==
  note: P_AEB_VSC_InterSwt4AcuteWarning
  AEB_VehResp : Inport
  FCW_AEB_VehFctSts : Inport  Port=2
  vehUnstableStateFlg : Inport  Port=3
  vehAssociatedSysStateSuppCond : Inport  Port=4
  "vehConfigFCW " : Inport  Port=5
  blockTimeWarning : Inport  Port=6
  Compare To Constant : simulink/Logic and Bit Operations/Compare To Constant  relop===  const=CNSwtOFF  OutDataTypeStr=boolean
  Compare To Constant1 : simulink/Logic and Bit Operations/Compare To Constant  relop===  const=CN_VSC_FCW_AEB_Error  OutDataTypeStr=boolean
  Compare To Constant2 : simulink/Logic and Bit Operations/Compare To Constant  relop===  const=CN_VSC_FCW_AEB_PerfmDgdtn  OutDataTypeStr=boolean
  Compare To Constant3 : simulink/Logic and Bit Operations/Compare To Constant  relop===  const=CNVehConfigFCWNone  OutDataTypeStr=boolean
  Compare To Constant4 : simulink/Logic and Bit Operations/Compare To Constant  relop=>  const=CNNull  OutDataTypeStr=boolean
  Compare To Constant5 : simulink/Logic and Bit Operations/Compare To Constant  relop===  const=TRUE  OutDataTypeStr=boolean
  Compare To Constant6 : simulink/Logic and Bit Operations/Compare To Constant  relop===  const=TRUE  OutDataTypeStr=boolean
  Compare To Constant7 : simulink/Logic and Bit Operations/Compare To Constant  relop===  const=CNSwtOFF  OutDataTypeStr=boolean
  Compare To Constant8 : simulink/Logic and Bit Operations/Compare To Constant  relop===  const=CN_VSC_FCW_AEB_TempUnAvlbl  OutDataTypeStr=boolean
  Constant : Constant  Value=P_AEB_InterSwt4AcuteWarning
  Logical Operator : Logic  Operator=OR  Inputs=3  AllPortsSameDT=off  OutDataTypeStr=boolean
  Logical Operator1 : Logic  Operator=OR  Inputs=7  AllPortsSameDT=off  OutDataTypeStr=boolean
  VSCvehAcuteWarningSuppCond : Outport
  wires:
  Compare To Constant8/1 -> Logical Operator/3
  Compare To Constant1/1 -> Logical Operator/1
  Compare To Constant2/1 -> Logical Operator/2
  Compare To Constant7/1 -> Logical Operator1/7
  Constant/1 -> Compare To Constant7/1
  Compare To Constant6/1 -> Logical Operator1/3
  Compare To Constant5/1 -> Logical Operator1/4
  Compare To Constant4/1 -> Logical Operator1/6
  "vehConfigFCW "/1 -> Compare To Constant3/1
  Logical Operator1/1 -> VSCvehAcuteWarningSuppCond/1
  vehUnstableStateFlg/1 -> Compare To Constant6/1
  Logical Operator/1 -> Logical Operator1/2
  blockTimeWarning/1 -> Compare To Constant4/1
  FCW_AEB_VehFctSts/1 -> Compare To Constant1/1, Compare To Constant2/1, Compare To Constant8/1
  vehAssociatedSysStateSuppCond/1 -> Compare To Constant5/1
  Compare To Constant3/1 -> Logical Operator1/5
  Compare To Constant/1 -> Logical Operator1/1
  AEB_VehResp/1 -> Compare To Constant/1

== system TosAeb/FcwAebJa_Step_sys/ALG_AEBSystem/ALG_AEBController/AEB_VehicleStateCalcApp/VehSuppAborCondtionCalc/EBASuppAborConditonCalc/VehStateCalcEBAAborCalc ==
  AEB_VehResp : Inport
  FCW_AEB_VehFctSts : Inport  Port=2
  vehAssociatedSysStateSuppCond : Inport  Port=3
  Compare To Constant : simulink/Logic and Bit Operations/Compare To Constant  relop===  const=CNSwtOFF  OutDataTypeStr=boolean
  Compare To Constant1 : simulink/Logic and Bit Operations/Compare To Constant  relop===  const=CN_VSC_FCW_AEB_Error  OutDataTypeStr=boolean
  Compare To Constant2 : simulink/Logic and Bit Operations/Compare To Constant  relop===  const=CN_VSC_FCW_AEB_PerfmDgdtn  OutDataTypeStr=boolean
  Compare To Constant5 : simulink/Logic and Bit Operations/Compare To Constant  relop===  const=TRUE  OutDataTypeStr=boolean
  Compare To Constant8 : simulink/Logic and Bit Operations/Compare To Constant  relop===  const=CN_VSC_FCW_AEB_TempUnAvlbl  OutDataTypeStr=boolean
  Logical Operator : Logic  Operator=OR  Inputs=3  AllPortsSameDT=off  OutDataTypeStr=boolean
  Logical Operator1 : Logic  Operator=OR  Inputs=3  AllPortsSameDT=off  OutDataTypeStr=boolean
  VSCvehEBAAborCond : Outport
  wires:
  FCW_AEB_VehFctSts/1 -> Compare To Constant1/1, Compare To Constant2/1, Compare To Constant8/1
  Compare To Constant8/1 -> Logical Operator/3
  Compare To Constant1/1 -> Logical Operator/1
  Compare To Constant2/1 -> Logical Operator/2
  Compare To Constant5/1 -> Logical Operator1/3
  vehAssociatedSysStateSuppCond/1 -> Compare To Constant5/1
  Logical Operator1/1 -> VSCvehEBAAborCond/1
  Logical Operator/1 -> Logical Operator1/2
  Compare To Constant/1 -> Logical Operator1/1
  AEB_VehResp/1 -> Compare To Constant/1

== system TosAeb/FcwAebJa_Step_sys/ALG_AEBSystem/ALG_AEBController/AEB_VehicleStateCalcApp/VehSuppAborCondtionCalc/EBASuppAborConditonCalc/VehStateCalcEBASuppCalc ==
  AEB_VehResp : Inport
  FCW_AEB_VehFctSts : Inport  Port=2
  vehUnstableStateFlg : Inport  Port=3
  vehAssociatedSysStateSuppCond : Inport  Port=4
  "vehConfigAEB " : Inport  Port=5
  blockTimeBrake : Inport  Port=6
  Compare To Constant : simulink/Logic and Bit Operations/Compare To Constant  relop===  const=CNSwtOFF  OutDataTypeStr=boolean
  Compare To Constant1 : simulink/Logic and Bit Operations/Compare To Constant  relop===  const=CN_VSC_FCW_AEB_Error  OutDataTypeStr=boolean
  Compare To Constant2 : simulink/Logic and Bit Operations/Compare To Constant  relop===  const=CN_VSC_FCW_AEB_PerfmDgdtn  OutDataTypeStr=boolean
  Compare To Constant3 : simulink/Logic and Bit Operations/Compare To Constant  relop===  const=CNVehConfigAEBNone  OutDataTypeStr=boolean
  Compare To Constant4 : simulink/Logic and Bit Operations/Compare To Constant  relop=>  const=CNNull  OutDataTypeStr=boolean
  Compare To Constant5 : simulink/Logic and Bit Operations/Compare To Constant  relop===  const=TRUE  OutDataTypeStr=boolean
  Compare To Constant6 : simulink/Logic and Bit Operations/Compare To Constant  relop===  const=TRUE  OutDataTypeStr=boolean
  Compare To Constant7 : simulink/Logic and Bit Operations/Compare To Constant  relop===  const=CNSwtOFF  OutDataTypeStr=boolean
  Compare To Constant8 : simulink/Logic and Bit Operations/Compare To Constant  relop===  const=CN_VSC_FCW_AEB_TempUnAvlbl  OutDataTypeStr=boolean
  Constant : Constant  Value=P_AEB_InterSwtSts4EBA
  Logical Operator : Logic  Operator=OR  Inputs=3  AllPortsSameDT=off  OutDataTypeStr=boolean
  Logical Operator1 : Logic  Operator=OR  Inputs=7  AllPortsSameDT=off  OutDataTypeStr=boolean
  VSCvehEBASuppCond : Outport
  wires:
  Logical Operator/1 -> Logical Operator1/2
  FCW_AEB_VehFctSts/1 -> Compare To Constant1/1, Compare To Constant2/1, Compare To Constant8/1
  Compare To Constant8/1 -> Logical Operator/3
  Compare To Constant1/1 -> Logical Operator/1
  Compare To Constant2/1 -> Logical Operator/2
  Compare To Constant7/1 -> Logical Operator1/7
  Constant/1 -> Compare To Constant7/1
  Compare To Constant6/1 -> Logical Operator1/3
  Compare To Constant5/1 -> Logical Operator1/4
  AEB_VehResp/1 -> Compare To Constant/1
  Compare To Constant/1 -> Logical Operator1/1
  Compare To Constant3/1 -> Logical Operator1/5
  vehAssociatedSysStateSuppCond/1 -> Compare To Constant5/1
  blockTimeBrake/1 -> Compare To Constant4/1
  vehUnstableStateFlg/1 -> Compare To Constant6/1
  Logical Operator1/1 -> VSCvehEBASuppCond/1
  "vehConfigAEB "/1 -> Compare To Constant3/1
  Compare To Constant4/1 -> Logical Operator1/6

== system TosAeb/FcwAebJa_Step_sys/ALG_AEBSystem/ALG_AEBController/AEB_VehicleStateCalcApp/VehSuppAborCondtionCalc/LatentWarningSuppAborConditonCalc/VehStateCalcLatentWarningAborCalc ==
  AEB_VehResp : Inport
  FCW_AEB_VehFctSts : Inport  Port=2
  vehAssociatedSysStateSuppCond : Inport  Port=3
  Compare To Constant : simulink/Logic and Bit Operations/Compare To Constant  relop===  const=CNSwtOFF  OutDataTypeStr=boolean
  Compare To Constant1 : simulink/Logic and Bit Operations/Compare To Constant  relop===  const=CN_VSC_FCW_AEB_Error  OutDataTypeStr=boolean
  Compare To Constant2 : simulink/Logic and Bit Operations/Compare To Constant  relop===  const=CN_VSC_FCW_AEB_PerfmDgdtn  OutDataTypeStr=boolean
  Compare To Constant5 : simulink/Logic and Bit Operations/Compare To Constant  relop===  const=TRUE  OutDataTypeStr=boolean
  Compare To Constant8 : simulink/Logic and Bit Operations/Compare To Constant  relop===  const=CN_VSC_FCW_AEB_TempUnAvlbl  OutDataTypeStr=boolean
  Logical Operator : Logic  Operator=OR  Inputs=3  AllPortsSameDT=off  OutDataTypeStr=boolean
  Logical Operator1 : Logic  Operator=OR  Inputs=3  AllPortsSameDT=off  OutDataTypeStr=boolean
  VSCvehLatentWarningAborCond : Outport
  wires:
  Compare To Constant8/1 -> Logical Operator/3
  Compare To Constant1/1 -> Logical Operator/1
  Compare To Constant2/1 -> Logical Operator/2
  Compare To Constant5/1 -> Logical Operator1/3
  vehAssociatedSysStateSuppCond/1 -> Compare To Constant5/1
  Logical Operator1/1 -> VSCvehLatentWarningAborCond/1
  Logical Operator/1 -> Logical Operator1/2
  FCW_AEB_VehFctSts/1 -> Compare To Constant1/1, Compare To Constant2/1, Compare To Constant8/1
  Compare To Constant/1 -> Logical Operator1/1
  AEB_VehResp/1 -> Compare To Constant/1
